simulink library "inp"  (Simulink 8.2)
settings: Solver=ode45  SolverMode=SingleTasking  StartTime=0.0  StopTime=10.0  RelTol=1e-3
undefined symbols (not defined in the file or in its folder): C_TICK_TIME_S, ENUM_FAULTSTATE_FAIL, ENUM_FAULTSTATE_INDETERMINATE, ENUM_FAULTSTATE_PASS, ENUM_KEYSWITCH_ON, FALSE, KINP_ABS1CANCommuOk_flg_ovrdflg, KINP_ABS1CANCommuOk_flg_ovrdval, KINP_ABS1CANPeriodTime_s, KINP_ABS1CANRolCntOk_flg_ovrdflg, KINP_ABS1CANRolCntOk_flg_ovrdval, KINP_ABS2CANChkSumOk_flg_ovrdflg, KINP_ABS2CANChkSumOk_flg_ovrdval, KINP_ABS2CANCommuOk_flg_ovrdflg, KINP_ABS2CANCommuOk_flg_ovrdval, KINP_ABS2CANPeriodTime_s, KINP_ABS2CANRolCntOk_flg_ovrdflg, KINP_ABS2CANRolCntOk_flg_ovrdval, KINP_ABS3CANCommuOk_flg_ovrdflg, KINP_ABS3CANCommuOk_flg_ovrdval, KINP_ABS4CANCommuOk_flg_ovrdflg, KINP_ABS4CANCommuOk_flg_ovrdval, KINP_ABSActive_flg_defval, KINP_ABSActive_flg_invalid, KINP_ABSActive_flg_ovrdflg, KINP_ABSActive_flg_ovrdval, KINP_ABSActive_flg_setvalid, KINP_ABSActive_flg_srvcflg, KINP_ABSActive_flg_srvcval, KINP_ABSCANAlive_flg_ovrdflg, KINP_ABSCANAlive_flg_ovrdval, KINP_ABSCANCommuOk_flg_ovrdflg, KINP_ABSCANCommuOk_flg_ovrdval, KINP_ABSCANValidOk_flg_ovrdflg, KINP_ABSCANValidOk_flg_ovrdval, KINP_ABSFault_flg_defval, KINP_ABSFault_flg_invalid, KINP_ABSFault_flg_ovrdflg, KINP_ABSFault_flg_ovrdval, KINP_ABSFault_flg_setvalid, KINP_ABSFault_flg_srvcflg, KINP_ABSFault_flg_srvcval, KINP_AC1CANCommuOk_flg_ovrdflg, KINP_AC1CANCommuOk_flg_ovrdval, KINP_ACCANAlive_flg_ovrdflg, KINP_ACCANAlive_flg_ovrdval, KINP_ACCANCommuOk_flg_ovrdflg, KINP_ACCANCommuOk_flg_ovrdval, KINP_ACCANValidOk_flg_ovrdflg, KINP_ACCANValidOk_flg_ovrdval, KINP_ACCCancelH_flg_defval, KINP_ACCCancelH_flg_invalid, KINP_ACCCancelH_flg_ovrdflg, KINP_ACCCancelH_flg_ovrdval, KINP_ACCCancelH_flg_setvalid, KINP_ACCCancelH_flg_srvcflg, KINP_ACCCancelH_flg_srvcval, KINP_AccelPedalPosn_pct_defval, KINP_AccelPedalPosn_pct_gain, KINP_AccelPedalPosn_pct_invalid ... (+1866)
libraries: inp x192, hcu_lib x34, simulink x33
(part 17 of 21)

== system inp/INP/EPM_signals/LaneMarkWidthLeft/min_max_limit ==
  in : Inport
  MinMax10 : MinMax  Function=max  Inputs=2
  MinMax5 : MinMax  Inputs=2
  maxval : Constant  Value=KINP_LaneMrkWidL_m_maxval
  minval : Constant  Value=KINP_LaneMrkWidL_m_minval
  out : Outport
  wires:
  MinMax5/1 -> MinMax10/1
  in/1 -> MinMax5/1
  MinMax10/1 -> out/1
  maxval/1 -> MinMax5/2
  minval/1 -> MinMax10/2

== system inp/INP/EPM_signals/LaneMarkWidthRight/conv_gain_offset ==
  in : Inport
  Product : Product  RndMeth=Zero
  Sum : Sum
  gain : Constant  Value=KINP_LaneMrkWidR_m_gain
  offset : Constant  Value=KINP_LaneMrkWidR_m_offset
  out : Outport
  wires:
  offset/1 -> Sum/2
  Product/1 -> Sum/1
  gain/1 -> Product/2
  in/1 -> Product/1
  Sum/1 -> out/1

== system inp/INP/EPM_signals/LaneMarkWidthRight/min_max_limit ==
  in : Inport
  MinMax10 : MinMax  Function=max  Inputs=2
  MinMax5 : MinMax  Inputs=2
  maxval : Constant  Value=KINP_LaneMrkWidR_m_maxval
  minval : Constant  Value=KINP_LaneMrkWidR_m_minval
  out : Outport
  wires:
  minval/1 -> MinMax10/2
  maxval/1 -> MinMax5/2
  MinMax10/1 -> out/1
  in/1 -> MinMax5/1
  MinMax5/1 -> MinMax10/1

== system inp/INP/EPM_signals/TimeToLaneCrossingLeft/conv_gain_offset ==
  in : Inport
  Product : Product  RndMeth=Zero
  Sum : Sum
  gain : Constant  Value=KINP_TLCL_s_gain
  offset : Constant  Value=KINP_TLCL_s_offset
  out : Outport
  wires:
  offset/1 -> Sum/2
  Product/1 -> Sum/1
  gain/1 -> Product/2
  in/1 -> Product/1
  Sum/1 -> out/1

== system inp/INP/EPM_signals/TimeToLaneCrossingLeft/min_max_limit ==
  in : Inport
  MinMax10 : MinMax  Function=max  Inputs=2
  MinMax5 : MinMax  Inputs=2
  maxval : Constant  Value=KINP_TLCL_s_maxval
  minval : Constant  Value=KINP_TLCL_s_minval
  out : Outport
  wires:
  minval/1 -> MinMax10/2
  maxval/1 -> MinMax5/2
  MinMax10/1 -> out/1
  in/1 -> MinMax5/1
  MinMax5/1 -> MinMax10/1

== system inp/INP/EPM_signals/TimeToLaneCrossingRight/conv_gain_offset ==
  in : Inport
  Product : Product  RndMeth=Zero
  Sum : Sum
  gain : Constant  Value=KINP_TLCR_s_gain
  offset : Constant  Value=KINP_TLCR_s_offset
  out : Outport
  wires:
  Sum/1 -> out/1
  in/1 -> Product/1
  gain/1 -> Product/2
  Product/1 -> Sum/1
  offset/1 -> Sum/2

== system inp/INP/EPM_signals/TimeToLaneCrossingRight/min_max_limit ==
  in : Inport
  MinMax10 : MinMax  Function=max  Inputs=2
  MinMax5 : MinMax  Inputs=2
  maxval : Constant  Value=KINP_TLCR_s_maxval
  minval : Constant  Value=KINP_TLCR_s_minval
  out : Outport
  wires:
  MinMax5/1 -> MinMax10/1
  in/1 -> MinMax5/1
  MinMax10/1 -> out/1
  maxval/1 -> MinMax5/2
  minval/1 -> MinMax10/2

== system inp/INP/EPM_signals/ViewRangeStartL/conv_gain_offset ==
  in : Inport
  Product : Product  RndMeth=Zero
  Sum : Sum
  gain : Constant  Value=KINP_ViewRangeStartL_m_gain
  offset : Constant  Value=KINP_ViewRangeStartL_m_offset
  out : Outport
  wires:
  Sum/1 -> out/1
  in/1 -> Product/1
  gain/1 -> Product/2
  Product/1 -> Sum/1
  offset/1 -> Sum/2

== system inp/INP/EPM_signals/ViewRangeStartL/min_max_limit ==
  in : Inport
  MinMax10 : MinMax  Function=max  Inputs=2
  MinMax5 : MinMax  Inputs=2
  maxval : Constant  Value=KINP_ViewRangeStartL_m_maxval
  minval : Constant  Value=KINP_ViewRangeStartL_m_minval
  out : Outport
  wires:
  MinMax5/1 -> MinMax10/1
  in/1 -> MinMax5/1
  MinMax10/1 -> out/1
  maxval/1 -> MinMax5/2
  minval/1 -> MinMax10/2

== system inp/INP/EPM_signals/ViewRangeStartR/conv_gain_offset ==
  in : Inport
  Product : Product  RndMeth=Zero
  Sum : Sum
  gain : Constant  Value=KINP_ViewRangeStartR_m_gain
  offset : Constant  Value=KINP_ViewRangeStartR_m_offset
  out : Outport
  wires:
  offset/1 -> Sum/2
  Product/1 -> Sum/1
  gain/1 -> Product/2
  in/1 -> Product/1
  Sum/1 -> out/1

== system inp/INP/EPM_signals/ViewRangeStartR/min_max_limit ==
  in : Inport
  MinMax10 : MinMax  Function=max  Inputs=2
  MinMax5 : MinMax  Inputs=2
  maxval : Constant  Value=KINP_ViewRangeStartR_m_maxval
  minval : Constant  Value=KINP_ViewRangeStartR_m_minval
  out : Outport
  wires:
  minval/1 -> MinMax10/2
  maxval/1 -> MinMax5/2
  MinMax10/1 -> out/1
  in/1 -> MinMax5/1
  MinMax5/1 -> MinMax10/1

== system inp/INP/EPS_signals/AckCurStrAngReq/conv_gain_offset ==
  in : Inport
  Product : Product  RndMeth=Zero
  Sum : Sum
  gain : Constant  Value=KINP_AckCurStrAngReq_deg_gain
  offset : Constant  Value=KINP_AckCurStrAngReq_deg_offset
  out : Outport
  wires:
  Sum/1 -> out/1
  in/1 -> Product/1
  gain/1 -> Product/2
  Product/1 -> Sum/1
  offset/1 -> Sum/2

== system inp/INP/EPS_signals/AckCurStrAngReq/min_max_limit ==
  in : Inport
  MinMax10 : MinMax  Function=max  Inputs=2
  MinMax5 : MinMax  Inputs=2
  maxval : Constant  Value=KINP_AckCurStrAngReq_deg_maxval
  minval : Constant  Value=KINP_AckCurStrAngReq_deg_minval
  out : Outport
  wires:
  MinMax5/1 -> MinMax10/1
  in/1 -> MinMax5/1
  MinMax10/1 -> out/1
  maxval/1 -> MinMax5/2
  minval/1 -> MinMax10/2

== system inp/INP/EPS_signals/AckCurStrTqReq/conv_gain_offset ==
  in : Inport
  Product : Product  RndMeth=Zero
  Sum : Sum
  gain : Constant  Value=KINP_AckCurStrTqReq_Nm_gain
  offset : Constant  Value=KINP_AckCurStrTqReq_Nm_offset
  out : Outport
  wires:
  offset/1 -> Sum/2
  Product/1 -> Sum/1
  gain/1 -> Product/2
  in/1 -> Product/1
  Sum/1 -> out/1

== system inp/INP/EPS_signals/AckCurStrTqReq/min_max_limit ==
  in : Inport
  MinMax10 : MinMax  Function=max  Inputs=2
  MinMax5 : MinMax  Inputs=2
  maxval : Constant  Value=KINP_AckCurStrTqReq_Nm_maxval
  minval : Constant  Value=KINP_AckCurStrTqReq_Nm_minval
  out : Outport
  wires:
  minval/1 -> MinMax10/2
  maxval/1 -> MinMax5/2
  MinMax10/1 -> out/1
  in/1 -> MinMax5/1
  MinMax5/1 -> MinMax10/1

== system inp/INP/EPS_signals/EPSBattVolt/conv_gain_offset ==
  in : Inport
  Product : Product  RndMeth=Zero
  Sum : Sum
  gain : Constant  Value=KINP_EPSBattVolt_V_gain
  offset : Constant  Value=KINP_EPSBattVolt_V_offset
  out : Outport
  wires:
  Sum/1 -> out/1
  in/1 -> Product/1
  gain/1 -> Product/2
  Product/1 -> Sum/1
  offset/1 -> Sum/2

== system inp/INP/EPS_signals/EPSBattVolt/min_max_limit ==
  in : Inport
  MinMax10 : MinMax  Function=max  Inputs=2
  MinMax5 : MinMax  Inputs=2
  maxval : Constant  Value=KINP_EPSBattVolt_V_maxval
  minval : Constant  Value=KINP_EPSBattVolt_V_minval
  out : Outport
  wires:
  MinMax5/1 -> MinMax10/1
  in/1 -> MinMax5/1
  MinMax10/1 -> out/1
  maxval/1 -> MinMax5/2
  minval/1 -> MinMax10/2

== system inp/INP/EPS_signals/EPSElectPWR/conv_gain_offset ==
  in : Inport
  Product : Product  RndMeth=Zero
  Sum : Sum
  gain : Constant  Value=KINP_EPSElectPWR_A_gain
  offset : Constant  Value=KINP_EPSElectPWR_A_offset
  out : Outport
  wires:
  offset/1 -> Sum/2
  Product/1 -> Sum/1
  gain/1 -> Product/2
  in/1 -> Product/1
  Sum/1 -> out/1

== system inp/INP/EPS_signals/EPSElectPWR/min_max_limit ==
  in : Inport
  MinMax10 : MinMax  Function=max  Inputs=2
  MinMax5 : MinMax  Inputs=2
  maxval : Constant  Value=KINP_EPSElectPWR_A_maxval
  minval : Constant  Value=KINP_EPSElectPWR_A_minval
  out : Outport
  wires:
  minval/1 -> MinMax10/2
  maxval/1 -> MinMax5/2
  MinMax10/1 -> out/1
  in/1 -> MinMax5/1
  MinMax5/1 -> MinMax10/1

== system inp/INP/EPS_signals/RelSteerAngle/conv_gain_offset ==
  in : Inport
  Product : Product  RndMeth=Zero
  Sum : Sum
  gain : Constant  Value=KINP_RelSteerAngle_deg_gain
  offset : Constant  Value=KINP_RelSteerAngle_deg_offset
  out : Outport
  wires:
  offset/1 -> Sum/2
  Product/1 -> Sum/1
  gain/1 -> Product/2
  in/1 -> Product/1
  Sum/1 -> out/1

== system inp/INP/EPS_signals/RelSteerAngle/min_max_limit ==
  in : Inport
  MinMax10 : MinMax  Function=max  Inputs=2
  MinMax5 : MinMax  Inputs=2
  maxval : Constant  Value=KINP_RelSteerAngle_deg_maxval
  minval : Constant  Value=KINP_RelSteerAngle_deg_minval
  out : Outport
  wires:
  minval/1 -> MinMax10/2
  maxval/1 -> MinMax5/2
  MinMax10/1 -> out/1
  in/1 -> MinMax5/1
  MinMax5/1 -> MinMax10/1

== system inp/INP/EPS_signals/SteerTorque/conv_gain_offset ==
  in : Inport
  Product : Product  RndMeth=Zero
  Sum : Sum
  gain : Constant  Value=KINP_SteerTorque_Nm_gain
  offset : Constant  Value=KINP_SteerTorque_Nm_offset
  out : Outport
  wires:
  Sum/1 -> out/1
  in/1 -> Product/1
  gain/1 -> Product/2
  Product/1 -> Sum/1
  offset/1 -> Sum/2

== system inp/INP/EPS_signals/SteerTorque/min_max_limit ==
  in : Inport
  MinMax10 : MinMax  Function=max  Inputs=2
  MinMax5 : MinMax  Inputs=2
  maxval : Constant  Value=KINP_SteerTorque_Nm_maxval
  minval : Constant  Value=KINP_SteerTorque_Nm_minval
  out : Outport
  wires:
  MinMax5/1 -> MinMax10/1
  in/1 -> MinMax5/1
  MinMax10/1 -> out/1
  maxval/1 -> MinMax5/2
  minval/1 -> MinMax10/2

== system inp/INP/EPS_signals/SteeringAngle/conv_gain_offset ==
  in : Inport
  Product : Product  RndMeth=Zero
  Sum : Sum
  gain : Constant  Value=KINP_SteerAngle_deg_gain
  offset : Constant  Value=KINP_SteerAngle_deg_offset
  out : Outport
  wires:
  offset/1 -> Sum/2
  Product/1 -> Sum/1
  gain/1 -> Product/2
  in/1 -> Product/1
  Sum/1 -> out/1

== system inp/INP/EPS_signals/SteeringAngle/min_max_limit ==
  in : Inport
  MinMax10 : MinMax  Function=max  Inputs=2
  MinMax5 : MinMax  Inputs=2
  maxval : Constant  Value=KINP_SteerAngle_deg_maxval
  minval : Constant  Value=KINP_SteerAngle_deg_minval
  out : Outport
  wires:
  minval/1 -> MinMax10/2
  maxval/1 -> MinMax5/2
  MinMax10/1 -> out/1
  in/1 -> MinMax5/1
  MinMax5/1 -> MinMax10/1

== system inp/INP/EPS_signals/SteeringSpeed/conv_gain_offset ==
  in : Inport
  Product : Product  RndMeth=Zero
  Sum : Sum
  gain : Constant  Value=KINP_SteerSpeed_degps_gain
  offset : Constant  Value=KINP_SteerSpeed_degps_offset
  out : Outport
  wires:
  Sum/1 -> out/1
  in/1 -> Product/1
  gain/1 -> Product/2
  Product/1 -> Sum/1
  offset/1 -> Sum/2

== system inp/INP/EPS_signals/SteeringSpeed/min_max_limit ==
  in : Inport
  MinMax10 : MinMax  Function=max  Inputs=2
  MinMax5 : MinMax  Inputs=2
  maxval : Constant  Value=KINP_SteerSpeed_degps_maxval
  minval : Constant  Value=KINP_SteerSpeed_degps_minval
  out : Outport
  wires:
  MinMax5/1 -> MinMax10/1
  in/1 -> MinMax5/1
  MinMax10/1 -> out/1
  maxval/1 -> MinMax5/2
  minval/1 -> MinMax10/2

== system inp/INP/HMI_signals/DefMaxACCSetSpeed/conv_gain_offset ==
  in : Inport
  Product : Product  RndMeth=Zero
  Sum : Sum
  gain : Constant  Value=KINP_DefMaxACCSetSpeed_kph_gain
  offset : Constant  Value=KINP_DefMaxACCSetSpeed_kph_offset
  out : Outport
  wires:
  offset/1 -> Sum/2
  Product/1 -> Sum/1
  gain/1 -> Product/2
  in/1 -> Product/1
  Sum/1 -> out/1

== system inp/INP/HMI_signals/DefMaxACCSetSpeed/min_max_limit ==
  in : Inport
  MinMax10 : MinMax  Function=max  Inputs=2
  MinMax5 : MinMax  Inputs=2
  maxval : Constant  Value=KINP_DefMaxACCSetSpeed_kph_maxval
  minval : Constant  Value=KINP_DefMaxACCSetSpeed_kph_minval
  out : Outport
  wires:
  minval/1 -> MinMax10/2
  maxval/1 -> MinMax5/2
  MinMax10/1 -> out/1
  in/1 -> MinMax5/1
  MinMax5/1 -> MinMax10/1

== system inp/INP/HWIO_signals/BatteryVolt/ad_signal_process ==
  raw_value : Inport
  raw_upper_limit : Inport  Port=2
  raw_lower_limit : Inport  Port=3
  adc_fault : Inport  Port=4
  vref_fault : Inport  Port=5
  ts : Inport  Port=6
  Active_fault_flg : Inport  Port=7
  tc : Inport  Port=8
  def_value : Inport  Port=9
  cal_ovrdflg : Inport  Port=10
  cal_ovrdval : Inport  Port=11
  service_flag : Inport  Port=12
  service_value : Inport  Port=13
  Logical Operator2 : Logic  Operator=NOT  AllPortsSameDT=off  OutDataTypeStr=boolean
  Relational Operator : RelationalOperator  Operator=<  OutDataTypeStr=boolean
  Relational Operator1 : RelationalOperator  Operator=<  OutDataTypeStr=boolean
  override signal : hcu_lib/override signal
  sig_valid_check : Subsystem  RTWFcnName=sig_valid_check
      settings: RTWFcnNameOpts=User specified
  signal_filter : Subsystem
  signal_range_high_flt : Outport
  signal_range_low_flt : Outport  Port=2
  valid_signal : Outport  Port=3
  signal_valid_flg : Outport  Port=4
  wires:
  Active_fault_flg/1 -> sig_valid_check/7(Active_fault_flg)
  Logical Operator2/1 -> override signal/1
  ts/1 -> signal_filter/3(Ts)
  tc/1 -> signal_filter/2(Tc)
  raw_value/1 -> [raw_value], signal_filter/1(raw_input)
  Relational Operator/1 -> sig_valid_check/4(range_high_ifault_flg)
  [raw_value] -> Relational Operator1/1, Relational Operator/2
  def_value/1 -> sig_valid_check/6(def_value)
  raw_lower_limit/1 -> Relational Operator1/2
  raw_upper_limit/1 -> Relational Operator/1
  sig_valid_check/3(fault_flg) -> Logical Operator2/1
  signal_filter/1(raw_input_filt) -> sig_valid_check/1(input_signal_filt)
  Relational Operator1/1 -> sig_valid_check/5(range_low_ifault_flg)
  sig_valid_check/1(range_high_flt) -> signal_range_high_flt/1
  sig_valid_check/2(range_low_flt) -> signal_range_low_flt/1
  service_flag/1 -> override signal/3
  service_value/1 -> override signal/4
  cal_ovrdflg/1 -> override signal/5
  cal_ovrdval/1 -> override signal/6
  sig_valid_check/4(valid_signal) -> override signal/2
  override signal/1 -> valid_signal/1
  override signal/2 -> signal_valid_flg/1
  adc_fault/1 -> sig_valid_check/2(adc_fault)
  vref_fault/1 -> sig_valid_check/3(vref_fault)

== system inp/INP/HWIO_signals/IgnitionVolt/ad_signal_process ==
  raw_value : Inport
  raw_upper_limit : Inport  Port=2
  raw_lower_limit : Inport  Port=3
  VHAL_KeySwitchStatus_enum : Inport  Port=4
  adc_fault : Inport  Port=5
  vref_fault : Inport  Port=6
  ts : Inport  Port=7
  Active_fault_flg : Inport  Port=8
  tc : Inport  Port=9
  def_value : Inport  Port=10
  cal_ovrdflg : Inport  Port=11
  cal_ovrdval : Inport  Port=12
  service_flag : Inport  Port=13
  service_value : Inport  Port=14
  Constant : Constant  Value=ENUM_KEYSWITCH_ON
  Logical Operator : Logic  AllPortsSameDT=off  OutDataTypeStr=boolean
  Logical Operator1 : Logic  AllPortsSameDT=off  OutDataTypeStr=boolean
  Logical Operator2 : Logic  Operator=NOT  AllPortsSameDT=off  OutDataTypeStr=boolean
  Relational Operator : RelationalOperator  Operator=<  OutDataTypeStr=boolean
  Relational Operator1 : RelationalOperator  Operator=<  OutDataTypeStr=boolean
  Relational Operator2 : RelationalOperator  Operator===  OutDataTypeStr=boolean
  override signal : hcu_lib/override signal
  sig_valid_check : Subsystem  RTWFcnName=sig_valid_check
      settings: RTWFcnNameOpts=User specified
  signal_filter : Subsystem
  signal_range_high_flt : Outport
  signal_range_low_flt : Outport  Port=2
  valid_signal : Outport  Port=3
  signal_valid_flg : Outport  Port=4
  wires:
  vref_fault/1 -> sig_valid_check/3(vref_fault)
  adc_fault/1 -> sig_valid_check/2(adc_fault)
  override signal/2 -> signal_valid_flg/1
  override signal/1 -> valid_signal/1
  sig_valid_check/4(valid_signal) -> override signal/2
  cal_ovrdval/1 -> override signal/6
  cal_ovrdflg/1 -> override signal/5
  service_value/1 -> override signal/4
  service_flag/1 -> override signal/3
  sig_valid_check/2(range_low_flt) -> signal_range_low_flt/1
  sig_valid_check/1(range_high_flt) -> signal_range_high_flt/1
  Relational Operator1/1 -> Logical Operator1/1
  signal_filter/1(raw_input_filt) -> sig_valid_check/1(input_signal_filt)
  sig_valid_check/3(fault_flg) -> Logical Operator2/1
  raw_upper_limit/1 -> Relational Operator/1
  raw_lower_limit/1 -> Relational Operator1/2
  def_value/1 -> sig_valid_check/6(def_value)
  [raw_value] -> Relational Operator/2, Relational Operator1/1
  Relational Operator/1 -> Logical Operator/1
  raw_value/1 -> signal_filter/1(raw_input), [raw_value]
  tc/1 -> signal_filter/2(Tc)
  ts/1 -> signal_filter/3(Ts)
  Logical Operator2/1 -> override signal/1
  Active_fault_flg/1 -> sig_valid_check/7(Active_fault_flg)
  VHAL_KeySwitchStatus_enum/1 -> Relational Operator2/1
  Constant/1 -> Relational Operator2/2
  Relational Operator2/1 -> [CAN_KeyOn]
  Logical Operator/1 -> sig_valid_check/4(range_high_ifault_flg)
  [CAN_KeyOn] -> Logical Operator/2
  Logical Operator1/1 -> sig_valid_check/5(range_low_ifault_flg)
  [CAN_KeyOn] -> Logical Operator1/2

== system inp/INP/InputSignals/Diagnosis/ABS_CANSig_Detection ==
  VINP_BrkLampOnSigOk_flg_InHAL : Inport
  VINP_VehSpdSigOk_flg_InHAL : Inport  Port=2
  VINP_YawRateSigOk_flg_InHAL : Inport  Port=3
  VINP_LatAccelSigOk_flg_InHAL : Inport  Port=4
  VINP_LongAccelSigOk_flg_InHAL : Inport  Port=5
  VINP_MCPressureSigOk_flg_InHAL : Inport  Port=6
  VINP_SurfaceGradeSigOk_flg_InHAL : Inport  Port=7
  VINP_BrakBoosterPresSigOk_flg_InHAL : Inport  Port=8
  VINP_LFWheelSpeedSigOk_flg_InHAL : Inport  Port=9
  VINP_LRWheelSpeedSigOk_flg_InHAL : Inport  Port=10
  VINP_RFWheelSpeedSigOk_flg_InHAL : Inport  Port=11
  VINP_RRWheelSpeedSigOk_flg_InHAL : Inport  Port=12
  Logical Operator{1..24} (24 alike) : Logic  Operator=NOT  AllPortsSameDT=off  OutDataTypeStr=boolean
  Switch{1..12} (12 alike) : Switch  Criteria=u2 ~= 0
  Terminator{1..12} (12 alike) : Terminator
  ovrdflg1 : Constant  Value=KINP_CANYawRateSigOk_flg_ovrdflg
  ovrdflg10 : Constant  Value=KINP_CANLRWhlSpdSigOk_flg_ovrdflg
  ovrdflg11 : Constant  Value=KINP_CANRFWhlSpdSigOk_flg_ovrdflg
  ovrdflg12 : Constant  Value=KINP_CANRRWhlSpdSigOk_flg_ovrdflg
  ovrdflg2 : Constant  Value=KINP_CANLatAccelSigOk_flg_ovrdflg
  ovrdflg3 : Constant  Value=KINP_CANVehSpdSigOk_flg_ovrdflg
  ovrdflg4 : Constant  Value=KINP_CANLongAccelSigOk_flg_ovrdflg
  ovrdflg5 : Constant  Value=KINP_CANBrkLampOnSigOk_flg_ovrdflg
  ovrdflg6 : Constant  Value=KINP_CANMCPressureSigOk_flg_ovrdflg
  ovrdflg7 : Constant  Value=KINP_CANSurfGradeSigOk_flg_ovrdflg
  ovrdflg8 : Constant  Value=KINP_CANBrakBoostPresSigOk_flg_ovrdflg
  ovrdflg9 : Constant  Value=KINP_CANLFWhlSpdSigOk_flg_ovrdflg
  ovrdval1 : Constant  Value=KINP_CANYawRateSigOk_flg_ovrdval
  ovrdval10 : Constant  Value=KINP_CANLRWhlSpdSigOk_flg_ovrdval
  ovrdval11 : Constant  Value=KINP_CANRFWhlSpdSigOk_flg_ovrdval
  ovrdval12 : Constant  Value=KINP_CANRRWhlSpdSigOk_flg_ovrdval
  ovrdval2 : Constant  Value=KINP_CANLatAccelSigOk_flg_ovrdval
  ovrdval3 : Constant  Value=KINP_CANVehSpdSigOk_flg_ovrdval
  ovrdval4 : Constant  Value=KINP_CANLongAccelSigOk_flg_ovrdval
  ovrdval5 : Constant  Value=KINP_CANBrkLampOnSigOk_flg_ovrdval
  ovrdval6 : Constant  Value=KINP_CANMCPressureSigOk_flg_ovrdval
  ovrdval7 : Constant  Value=KINP_CANSurfGradeSigOk_flg_ovrdval
  ovrdval8 : Constant  Value=KINP_CANBrakBoostPresSigOk_flg_ovrdval
  ovrdval9 : Constant  Value=KINP_CANLFWhlSpdSigOk_flg_ovrdval
  wires:
  Logical Operator2/1 -> Terminator1/1  "VINP_CANYawRateSigFault_flg"
  Logical Operator3/1 -> Switch1/2
  ovrdval1/1 -> Switch1/3
  ovrdflg1/1 -> Logical Operator3/1
  Switch1/1 -> Logical Operator2/1
  VINP_YawRateSigOk_flg_InHAL/1 -> Switch1/1
  Logical Operator19/1 -> Terminator5/1  "VINP_CANVehSpdSigFault_flg"
  Logical Operator1/1 -> Terminator2/1  "VINP_CANBrkLampOnSigFault_flg"
  Logical Operator11/1 -> Switch3/2
  ovrdval5/1 -> Switch3/3
  ovrdflg5/1 -> Logical Operator11/1
  Switch3/1 -> Logical Operator1/1
  Logical Operator15/1 -> Switch7/2
  ovrdval3/1 -> Switch7/3
  ovrdflg3/1 -> Logical Operator15/1
  Switch7/1 -> Logical Operator19/1
  VINP_BrkLampOnSigOk_flg_InHAL/1 -> Switch3/1
  VINP_VehSpdSigOk_flg_InHAL/1 -> Switch7/1
  Logical Operator4/1 -> Terminator3/1  "VINP_CANLatAccelSigFault_flg"
  Logical Operator5/1 -> Switch2/2
  ovrdval2/1 -> Switch2/3
  ovrdflg2/1 -> Logical Operator5/1
  Switch2/1 -> Logical Operator4/1
  VINP_LatAccelSigOk_flg_InHAL/1 -> Switch2/1
  Logical Operator6/1 -> Terminator4/1  "VINP_CANLongAccelSigFault_flg"
  Logical Operator7/1 -> Switch4/2
  ovrdval4/1 -> Switch4/3
  ovrdflg4/1 -> Logical Operator7/1
  Switch4/1 -> Logical Operator6/1
  VINP_LongAccelSigOk_flg_InHAL/1 -> Switch4/1
  Logical Operator8/1 -> Terminator6/1  "VINP_CANMCPressureSigFault_flg"
  Logical Operator9/1 -> Switch5/2
  ovrdval6/1 -> Switch5/3
  ovrdflg6/1 -> Logical Operator9/1
  Switch5/1 -> Logical Operator8/1
  VINP_MCPressureSigOk_flg_InHAL/1 -> Switch5/1
  Logical Operator10/1 -> Terminator7/1  "VINP_CANSurfGradeSigFault_flg"
  Logical Operator12/1 -> Switch6/2
  ovrdval7/1 -> Switch6/3
  ovrdflg7/1 -> Logical Operator12/1
  Switch6/1 -> Logical Operator10/1
  VINP_SurfaceGradeSigOk_flg_InHAL/1 -> Switch6/1
  Logical Operator13/1 -> Terminator8/1  "VINP_CANBrakBoostPresSigFault_flg"
  Logical Operator14/1 -> Switch8/2
  ovrdval8/1 -> Switch8/3
  ovrdflg8/1 -> Logical Operator14/1
  Switch8/1 -> Logical Operator13/1
  VINP_BrakBoosterPresSigOk_flg_InHAL/1 -> Switch8/1
  Logical Operator16/1 -> Terminator9/1  "VINP_CANLFWhlSpdSigFault_flg"
  Logical Operator17/1 -> Switch9/2
  ovrdval9/1 -> Switch9/3
  ovrdflg9/1 -> Logical Operator17/1
  Switch9/1 -> Logical Operator16/1
  VINP_LFWheelSpeedSigOk_flg_InHAL/1 -> Switch9/1
  Logical Operator18/1 -> Terminator10/1  "VINP_CANLRWhlSpdSigFault_flg"
  Logical Operator20/1 -> Switch10/2
  ovrdval10/1 -> Switch10/3
  ovrdflg10/1 -> Logical Operator20/1
  Switch10/1 -> Logical Operator18/1
  VINP_LRWheelSpeedSigOk_flg_InHAL/1 -> Switch10/1
  Logical Operator21/1 -> Terminator11/1  "VINP_CANRFWhlSpdSigFault_flg"
  Logical Operator22/1 -> Switch11/2
  ovrdval11/1 -> Switch11/3
  ovrdflg11/1 -> Logical Operator22/1
  Switch11/1 -> Logical Operator21/1
  VINP_RFWheelSpeedSigOk_flg_InHAL/1 -> Switch11/1
  Logical Operator23/1 -> Terminator12/1  "VINP_CANRRWhlSpdSigFault_flg"
  Logical Operator24/1 -> Switch12/2
  ovrdval12/1 -> Switch12/3
  ovrdflg12/1 -> Logical Operator24/1
  Switch12/1 -> Logical Operator23/1
  VINP_RRWheelSpeedSigOk_flg_InHAL/1 -> Switch12/1

== system inp/INP/InputSignals/Diagnosis/AC_CANSig_Detection ==
  VINP_OutsideTempSigOk_flg_InHAL : Inport
  Logical Operator6 : Logic  Operator=NOT  AllPortsSameDT=off  OutDataTypeStr=boolean
  Logical Operator8 : Logic  Operator=NOT  AllPortsSameDT=off  OutDataTypeStr=boolean
  Switch2 : Switch  Criteria=u2 ~= 0
  Terminator : Terminator
  ovrdflg : Constant  Value=KINP_CANOutsideTempSigOk_flg_ovrdflg
  ovrdval : Constant  Value=KINP_CANOutsideTempSigOk_flg_ovrdval
  wires:
  VINP_OutsideTempSigOk_flg_InHAL/1 -> Switch2/1
  Switch2/1 -> Logical Operator6/1
  ovrdflg/1 -> Logical Operator8/1
  ovrdval/1 -> Switch2/3
  Logical Operator8/1 -> Switch2/2
  Logical Operator6/1 -> Terminator/1  "VINP_CANOutsideTempSigFault_flg"

== system inp/INP/InputSignals/Diagnosis/BCM_CANSig_Detection ==
  VINP_VehCrashedSigOk_flg_InHAL : Inport
  Logical Operator6 : Logic  Operator=NOT  AllPortsSameDT=off  OutDataTypeStr=boolean
  Logical Operator8 : Logic  Operator=NOT  AllPortsSameDT=off  OutDataTypeStr=boolean
  Switch2 : Switch  Criteria=u2 ~= 0
  Terminator : Terminator
  ovrdflg : Constant  Value=KINP_CANVehCrashdSigOk_flg_ovrdflg
  ovrdval : Constant  Value=KINP_CANVehCrashdSigOk_flg_ovrdval
  wires:
  Logical Operator6/1 -> Terminator/1  "VINP_CANVehCrashdSigFault_flg"
  Logical Operator8/1 -> Switch2/2
  ovrdval/1 -> Switch2/3
  ovrdflg/1 -> Logical Operator8/1
  Switch2/1 -> Logical Operator6/1
  VINP_VehCrashedSigOk_flg_InHAL/1 -> Switch2/1

== system inp/INP/InputSignals/Diagnosis/CANCommunicationDetection ==
  VINP_CANMsgABS1CommuOk_flg : Inport
  VINP_CANMsgABS2CommuOk_flg : Inport  Port=2
  VINP_CANMsgABS3CommuOk_flg : Inport  Port=3
  VINP_CANMsgABS4CommuOk_flg : Inport  Port=4
  VINP_CANMsgBCM1CommuOk_flg : Inport  Port=5
  VINP_CANMsgBCM2CommuOk_flg : Inport  Port=6
  VINP_CANMsgECM1CommuOk_flg : Inport  Port=7
  VINP_CANMsgECM2CommuOk_flg : Inport  Port=8
  VINP_CANMsgECM3CommuOk_flg : Inport  Port=9
  VINP_CANMsgECM9CommuOk_flg : Inport  Port=10
  VINP_CANMsgTCM1CommuOk_flg : Inport  Port=11
  VINP_CANMsgTCM2CommuOk_flg : Inport  Port=12
  VINP_CANMsgSAS1CommuOk_flg : Inport  Port=13
  VINP_CANMsgEPAS1CommuOk_flg : Inport  Port=14
  VINP_CANMsgEPAS2CommuOk_flg : Inport  Port=15
  VINP_CANMsgEPAS3CommuOk_flg : Inport  Port=16
  VINP_CANMsgMETER1CommuOk_flg : Inport  Port=17
  VINP_CANMsgMETER3CommuOk_flg : Inport  Port=18
  VINP_CANMsgAC1CommuOk_flg : Inport  Port=19
  VINP_CANMsgEPB1CommuOk_flg : Inport  Port=20
  VINP_CANMsgVCU1CommuOk_flg : Inport  Port=21
  VINP_CANMsgVCU2CommuOk_flg : Inport  Port=22
  VINP_CANMsgHCU3CommuOk_flg : Inport  Port=23
  VINP_CANMsgHCU4CommuOk_flg : Inport  Port=24
  VINP_CANMsgHMI2CommuOk_flg : Inport  Port=25
  VINP_CANMsgHMI1CommuOk_flg : Inport  Port=26
  VINP_CANMsgCSW1CommuOk_flg : Inport  Port=27
  VINP_CANMsgEPMCommuOk_flg : Inport  Port=28
  Bus Selector : BusSelector  OutputSignals=VINP_CANMsgEPM1CommuOk_flg,VINP_CANMsgEPM2CommuOk_flg,VINP_CANMsgEPM3CommuOk_flg,VINP_CANMsgEPM4CommuOk_flg,VINP_CANMsgEPM5CommuOk_flg,VINP_CANMsgEPM6CommuOk_flg,VINP_CANMsgEPM7CommuOk_flg,VINP_CANMsgEPM8CommuOk_flg,VINP_CANMsgEPM9CommuOk_flg,VINP_CANMsgEPM10CommuOk_flg,VINP_CANMsgEPM11CommuOk_flg,VINP_CANMsgEPM12CommuOk_flg,VINP_CANMsgEPM13CommuOk_flg,VINP_CANMsgEPM14CommuOk_flg,VINP_CANMsgEPM15CommuOk_flg,VINP_CANMsgEPM16CommuOk_flg,VINP_CANMsgEPM17CommuOk_flg,VINP_CANMsgEPM18CommuOk_flg,VINP_CANMsgEPM19CommuOk_flg,VINP_CANMsgEPM20CommuOk_flg,VINP_CANMsgEPM21CommuOk_flg,VINP_CANMsgEPM22CommuOk_flg,VINP_CANMsgEPM23CommuOk_flg,VINP_CANMsgEPM24CommuOk_flg,VINP_CANMsgEPM25CommuOk_flg,VINP_CANMsgEPM26CommuOk_flg,VINP_CANMsgEPM27CommuOk_flg,VINP_CANMsgEPM28CommuOk_flg,VINP_CANMsgEPM29CommuOk_flg,VINP_CANMsgEPM30CommuOk_flg,VINP_CANMsgEPM31CommuOk_flg,VINP_CANMsgEPM32CommuOk_flg,VINP_CANMsgEPM33CommuOk_flg,VINP_CANMsgEPM34CommuOk_flg,VINP_CANMsgEPM35CommuOk_flg
  Logical Operator{1,6,8..12,14..21,23,25..27,29,31..32,34..35} (24 alike) : Logic  Operator=NOT  AllPortsSameDT=off  OutDataTypeStr=boolean
  Logical Operator13 : Logic  AllPortsSameDT=off  OutDataTypeStr=boolean
  Logical Operator2 : Logic  AllPortsSameDT=off  OutDataTypeStr=boolean
  Logical Operator{22,24,30,36} (4 alike) : Logic  Inputs=1  AllPortsSameDT=off  OutDataTypeStr=boolean
  Logical Operator28 : Logic  Inputs=5  AllPortsSameDT=off  OutDataTypeStr=boolean
  Logical Operator3 : Logic  AllPortsSameDT=off  OutDataTypeStr=boolean
  Logical Operator33 : Logic  Inputs=35  AllPortsSameDT=off  OutDataTypeStr=boolean
  Logical Operator4 : Logic  Inputs=4  AllPortsSameDT=off  OutDataTypeStr=boolean
  Logical Operator5 : Logic  Inputs=4  AllPortsSameDT=off  OutDataTypeStr=boolean
  Logical Operator7 : Logic  Inputs=4  AllPortsSameDT=off  OutDataTypeStr=boolean
  Switch{1..12} (12 alike) : Switch  Criteria=u2 ~= 0
  Terminator{,1..11} (12 alike) : Terminator
  ovrdflg : Constant  Value=KINP_ABSCANCommuOk_flg_ovrdflg
  ovrdflg1 : Constant  Value=KINP_ACCANCommuOk_flg_ovrdflg
  ovrdflg10 : Constant  Value=KINP_EPMCANCommuOk_flg_ovrdflg
  ovrdflg11 : Constant  Value=KINP_CSWCANCommuOk_flg_ovrdflg
  ovrdflg2 : Constant  Value=KINP_BCMCANCommuOk_flg_ovrdflg
  ovrdflg3 : Constant  Value=KINP_EPSCANCommuOk_flg_ovrdflg
  ovrdflg4 : Constant  Value=KINP_EPBCANCommuOk_flg_ovrdflg
  ovrdflg5 : Constant  Value=KINP_ECMCANCommuOk_flg_ovrdflg
  ovrdflg6 : Constant  Value=KINP_METERCANCommuOk_flg_ovrdflg
  ovrdflg7 : Constant  Value=KINP_VCUCANCommuOk_flg_ovrdflg
  ovrdflg8 : Constant  Value=KINP_TCMCANCommuOk_flg_ovrdflg
  ovrdflg9 : Constant  Value=KINP_HMICANCommuOk_flg_ovrdflg
  ovrdval : Constant  Value=KINP_ABSCANCommuOk_flg_ovrdval
  ovrdval1 : Constant  Value=KINP_ACCANCommuOk_flg_ovrdval
  ovrdval10 : Constant  Value=KINP_EPMCANCommuOk_flg_ovrdval
  ovrdval11 : Constant  Value=KINP_CSWCANCommuOk_flg_ovrdval
  ovrdval2 : Constant  Value=KINP_BCMCANCommuOk_flg_ovrdval
  ovrdval3 : Constant  Value=KINP_EPSCANCommuOk_flg_ovrdval
  ovrdval4 : Constant  Value=KINP_EPBCANCommuOk_flg_ovrdval
  ovrdval5 : Constant  Value=KINP_ECMCANCommuOk_flg_ovrdval
  ovrdval6 : Constant  Value=KINP_METERCANCommuOk_flg_ovrdval
  ovrdval7 : Constant  Value=KINP_VCUCANCommuOk_flg_ovrdval
  ovrdval8 : Constant  Value=KINP_TCMCANCommuOk_flg_ovrdval
  ovrdval9 : Constant  Value=KINP_HMICANCommuOk_flg_ovrdval
  VINP_ABSCANValid_flg_InProc : Outport
  VINP_BCMCANValid_flg_InProc : Outport  Port=2
  VINP_ECMCANValid_flg_InProc : Outport  Port=3
  VINP_TCMCANValid_flg_InProc : Outport  Port=4
  VINP_EPSCANValid_flg_InProc : Outport  Port=5
  VINP_METERCANValid_flg_InProc : Outport  Port=6
  VINP_ACCANValid_flg_InProc : Outport  Port=7
  VINP_EPBCANValid_flg_InProc : Outport  Port=8
  VINP_VCUCANValid_flg_InProc : Outport  Port=9
  VINP_HMICANValid_flg_InProc : Outport  Port=10
  VINP_CSWCANValid_flg_InProc : Outport  Port=11
  VINP_EPMCANValid_flg_InProc : Outport  Port=12
  wires:
  VINP_CANMsgSAS1CommuOk_flg/1 -> Logical Operator7/1
  Switch7/1 -> VINP_EPSCANValid_flg_InProc/1, Logical Operator19/1  "VINP_EPSCANValid_flg_InProc"
  ovrdflg3/1 -> Logical Operator15/1
  ovrdval3/1 -> Switch7/3
  Logical Operator7/1 -> Switch7/1
  Logical Operator15/1 -> Switch7/2
  VINP_CANMsgTCM1CommuOk_flg/1 -> Logical Operator3/1
  Switch2/1 -> VINP_ABSCANValid_flg_InProc/1, Logical Operator6/1  "VINP_ABSCANValid_flg_InProc"
  VINP_CANMsgBCM1CommuOk_flg/1 -> Logical Operator2/1
  Switch1/1 -> VINP_BCMCANValid_flg_InProc/1, Logical Operator9/1  "VINP_BCMCANValid_flg_InProc"
  Switch3/1 -> VINP_ECMCANValid_flg_InProc/1, Logical Operator1/1  "VINP_ECMCANValid_flg_InProc"
  Switch4/1 -> VINP_TCMCANValid_flg_InProc/1, Logical Operator17/1  "VINP_TCMCANValid_flg_InProc"
  ovrdflg/1 -> Logical Operator8/1
  ovrdval/1 -> Switch2/3
  ovrdflg2/1 -> Logical Operator10/1
  ovrdval2/1 -> Switch1/3
  ovrdflg5/1 -> Logical Operator11/1
  ovrdval5/1 -> Switch3/3
  ovrdflg8/1 -> Logical Operator12/1
  ovrdval8/1 -> Switch4/3
  Logical Operator4/1 -> Switch2/1
  Logical Operator2/1 -> Switch1/1
  Logical Operator5/1 -> Switch3/1
  Logical Operator3/1 -> Switch4/1
  Logical Operator8/1 -> Switch2/2
  Logical Operator10/1 -> Switch1/2
  Logical Operator11/1 -> Switch3/2
  Logical Operator12/1 -> Switch4/2
  Logical Operator6/1 -> Terminator/1  "VINP_ABSCANCommuFault_flg"
  Logical Operator9/1 -> Terminator1/1  "VINP_BCMCANCommuFault_flg"
  Logical Operator1/1 -> Terminator2/1  "VINP_ECMCANCommuFault_flg"
  Logical Operator17/1 -> Terminator3/1  "VINP_TCMCANCommuFault_flg"
  Logical Operator19/1 -> Terminator5/1  "VINP_EPSCANCommuFault_flg"
  Switch8/1 -> Logical Operator21/1, VINP_METERCANValid_flg_InProc/1  "VINP_METERCANValid_flg_InProc"
  ovrdflg6/1 -> Logical Operator20/1
  ovrdval6/1 -> Switch8/3
  Logical Operator13/1 -> Switch8/1
  Logical Operator20/1 -> Switch8/2
  Logical Operator21/1 -> Terminator6/1  "VINP_METERCANCommuFault_flg"
  VINP_CANMsgBCM2CommuOk_flg/1 -> Logical Operator2/2
  VINP_CANMsgTCM2CommuOk_flg/1 -> Logical Operator3/2
  VINP_CANMsgMETER1CommuOk_flg/1 -> Logical Operator13/1
  VINP_CANMsgMETER3CommuOk_flg/1 -> Logical Operator13/2
  Switch5/1 -> VINP_ACCANValid_flg_InProc/1, Logical Operator18/1  "VINP_ACCANValid_flg_InProc"
  ovrdflg1/1 -> Logical Operator16/1
  ovrdval1/1 -> Switch5/3
  Logical Operator22/1 -> Switch5/1
  Logical Operator16/1 -> Switch5/2
  Logical Operator18/1 -> Terminator4/1  "VINP_ACCANCommuFault_flg"
  VINP_CANMsgAC1CommuOk_flg/1 -> Logical Operator22/1
  Switch6/1 -> Logical Operator23/1, VINP_EPBCANValid_flg_InProc/1  "VINP_EPBCANValid_flg_InProc"
  ovrdflg4/1 -> Logical Operator14/1
  ovrdval4/1 -> Switch6/3
  Logical Operator24/1 -> Switch6/1
  Logical Operator14/1 -> Switch6/2
  Logical Operator23/1 -> Terminator7/1  "VINP_EPBCANCommuFault_flg"
  VINP_CANMsgEPB1CommuOk_flg/1 -> Logical Operator24/1
  Switch9/1 -> VINP_VCUCANValid_flg_InProc/1, Logical Operator26/1  "VINP_VCUCANValid_flg_InProc"
  ovrdflg7/1 -> Logical Operator25/1
  ovrdval7/1 -> Switch9/3
  Logical Operator28/1 -> Switch9/1
  Logical Operator25/1 -> Switch9/2
  Logical Operator26/1 -> Terminator8/1  "VINP_VCUCANCommuFault_flg"
  VINP_CANMsgVCU1CommuOk_flg/1 -> Logical Operator28/1
  VINP_CANMsgVCU2CommuOk_flg/1 -> Logical Operator28/2
  VINP_CANMsgHCU3CommuOk_flg/1 -> Logical Operator28/3
  VINP_CANMsgABS1CommuOk_flg/1 -> Logical Operator4/1
  VINP_CANMsgABS2CommuOk_flg/1 -> Logical Operator4/2
  VINP_CANMsgABS3CommuOk_flg/1 -> Logical Operator4/3
  VINP_CANMsgABS4CommuOk_flg/1 -> Logical Operator4/4
  VINP_CANMsgECM1CommuOk_flg/1 -> Logical Operator5/1
  VINP_CANMsgECM2CommuOk_flg/1 -> Logical Operator5/2
  VINP_CANMsgECM3CommuOk_flg/1 -> Logical Operator5/3
  VINP_CANMsgECM9CommuOk_flg/1 -> Logical Operator5/4
  VINP_CANMsgHCU4CommuOk_flg/1 -> Logical Operator28/4
  VINP_CANMsgHMI2CommuOk_flg/1 -> Logical Operator28/5
  Switch10/1 -> VINP_HMICANValid_flg_InProc/1, Logical Operator29/1  "VINP_HMICANValid_flg_InProc"
  ovrdflg9/1 -> Logical Operator27/1
  ovrdval9/1 -> Switch10/3
  Logical Operator30/1 -> Switch10/1
  Logical Operator27/1 -> Switch10/2
  Logical Operator29/1 -> Terminator9/1  "VINP_HMICANCommuFault_flg"
  VINP_CANMsgHMI1CommuOk_flg/1 -> Logical Operator30/1
  Switch11/1 -> VINP_EPMCANValid_flg_InProc/1, Logical Operator32/1  "VINP_EPMCANValid_flg_InProc"
  ovrdflg10/1 -> Logical Operator31/1
  ovrdval10/1 -> Switch11/3
  Logical Operator33/1 -> Switch11/1
  Logical Operator31/1 -> Switch11/2
  Logical Operator32/1 -> Terminator10/1  "VINP_EPMCANCommuFault_flg"
  VINP_CANMsgEPAS1CommuOk_flg/1 -> Logical Operator7/2
  VINP_CANMsgEPAS2CommuOk_flg/1 -> Logical Operator7/3
  VINP_CANMsgEPAS3CommuOk_flg/1 -> Logical Operator7/4
  Switch12/1 -> Logical Operator35/1, VINP_CSWCANValid_flg_InProc/1  "VINP_CSWCANValid_flg_InProc"
  ovrdflg11/1 -> Logical Operator34/1
  ovrdval11/1 -> Switch12/3
  Logical Operator36/1 -> Switch12/1
  Logical Operator34/1 -> Switch12/2
  Logical Operator35/1 -> Terminator11/1  "VINP_CSWCANCommuFault_flg"
  VINP_CANMsgCSW1CommuOk_flg/1 -> Logical Operator36/1
  VINP_CANMsgEPMCommuOk_flg/1 -> Bus Selector/1
  Bus Selector/21 -> Logical Operator33/21  "<VINP_CANMsgEPM21CommuOk_flg>"
  Bus Selector/35 -> Logical Operator33/35  "<VINP_CANMsgEPM35CommuOk_flg>"
  Bus Selector/24 -> Logical Operator33/24  "<VINP_CANMsgEPM24CommuOk_flg>"
  Bus Selector/29 -> Logical Operator33/29  "<VINP_CANMsgEPM29CommuOk_flg>"
  Bus Selector/34 -> Logical Operator33/34  "<VINP_CANMsgEPM34CommuOk_flg>"
  Bus Selector/22 -> Logical Operator33/22  "<VINP_CANMsgEPM22CommuOk_flg>"
  Bus Selector/20 -> Logical Operator33/20  "<VINP_CANMsgEPM20CommuOk_flg>"
  Bus Selector/25 -> Logical Operator33/25  "<VINP_CANMsgEPM25CommuOk_flg>"
  Bus Selector/23 -> Logical Operator33/23  "<VINP_CANMsgEPM23CommuOk_flg>"
  Bus Selector/33 -> Logical Operator33/33  "<VINP_CANMsgEPM33CommuOk_flg>"
  Bus Selector/28 -> Logical Operator33/28  "<VINP_CANMsgEPM28CommuOk_flg>"
  Bus Selector/26 -> Logical Operator33/26  "<VINP_CANMsgEPM26CommuOk_flg>"
  Bus Selector/27 -> Logical Operator33/27  "<VINP_CANMsgEPM27CommuOk_flg>"
  Bus Selector/32 -> Logical Operator33/32  "<VINP_CANMsgEPM32CommuOk_flg>"
  Bus Selector/30 -> Logical Operator33/30  "<VINP_CANMsgEPM30CommuOk_flg>"
  Bus Selector/31 -> Logical Operator33/31  "<VINP_CANMsgEPM31CommuOk_flg>"
  Bus Selector/16 -> Logical Operator33/16  "<VINP_CANMsgEPM16CommuOk_flg>"
  Bus Selector/6 -> Logical Operator33/6  "<VINP_CANMsgEPM6CommuOk_flg>"
  Bus Selector/2 -> Logical Operator33/2  "<VINP_CANMsgEPM2CommuOk_flg>"
  Bus Selector/4 -> Logical Operator33/4  "<VINP_CANMsgEPM4CommuOk_flg>"
  Bus Selector/17 -> Logical Operator33/17  "<VINP_CANMsgEPM17CommuOk_flg>"
  Bus Selector/5 -> Logical Operator33/5  "<VINP_CANMsgEPM5CommuOk_flg>"
  Bus Selector/14 -> Logical Operator33/14  "<VINP_CANMsgEPM14CommuOk_flg>"
  Bus Selector/1 -> Logical Operator33/1  "<VINP_CANMsgEPM1CommuOk_flg>"
  Bus Selector/13 -> Logical Operator33/13  "<VINP_CANMsgEPM13CommuOk_flg>"
  Bus Selector/19 -> Logical Operator33/19  "<VINP_CANMsgEPM19CommuOk_flg>"
  Bus Selector/3 -> Logical Operator33/3  "<VINP_CANMsgEPM3CommuOk_flg>"
  Bus Selector/12 -> Logical Operator33/12  "<VINP_CANMsgEPM12CommuOk_flg>"
  Bus Selector/9 -> Logical Operator33/9  "<VINP_CANMsgEPM9CommuOk_flg>"
  Bus Selector/8 -> Logical Operator33/8  "<VINP_CANMsgEPM8CommuOk_flg>"
  Bus Selector/7 -> Logical Operator33/7  "<VINP_CANMsgEPM7CommuOk_flg>"
  Bus Selector/15 -> Logical Operator33/15  "<VINP_CANMsgEPM15CommuOk_flg>"
  Bus Selector/11 -> Logical Operator33/11  "<VINP_CANMsgEPM11CommuOk_flg>"
  Bus Selector/10 -> Logical Operator33/10  "<VINP_CANMsgEPM10CommuOk_flg>"
  Bus Selector/18 -> Logical Operator33/18  "<VINP_CANMsgEPM18CommuOk_flg>"
